simulink model "rosbagData"  (R2023b)
settings: Solver=VariableStepAuto  StartTime=0.0  StopTime=625  RelTol=1e-3
model workspace (MAT data in the model file):
  Logfile_Data_Object : robotics.ros.BagSelection (value not decoded)
  Logfile_Path = '/Users/umphaipunatipunumphai/Downloads/2023_11_30_19_15_04_2T3MWRFVXLW056972fourwheelscontroller.bag'
  Logfile_Playback_Duration = nan
  Logfile_Playback_Offset = nan
libraries: robotlib x2
settings Model: Creator=umphaipunatipunumphai  LastModifiedBy=umphaipunatipunumphai

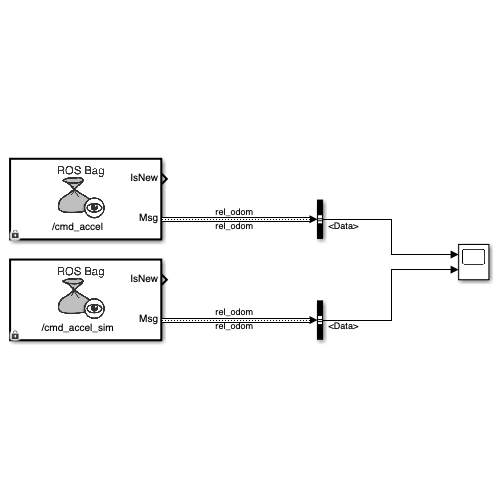
[thumbnail: Simulink's stored preview of the top-level diagram]
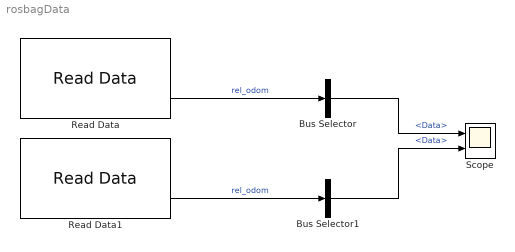
== system rosbagData ==
  Bus Selector : BusSelector  OutputSignals=Data
  Bus Selector1 : BusSelector  OutputSignals=Data
  Read Data : robotlib/Read Data  topic=/cmd_accel  sampleTime=-1  logfileType=rosbag  modelName=untitled  msgType=std_msgs/Float64
  Read Data1 : robotlib/Read Data  topic=/cmd_accel_sim  sampleTime=-1  logfileType=rosbag  modelName=untitled  msgType=std_msgs/Float64
  Scope : Scope  NumInputPorts=2
  wires:
  Read Data/2 -> Bus Selector/1  "rel_odom"
  Bus Selector/1 -> Scope/1  "<Data>"
  Read Data1/2 -> Bus Selector1/1  "rel_odom"
  Bus Selector1/1 -> Scope/2  "<Data>"
